simulink model "fft_core"  (Simulink 8.0) (continued)
== system fft_core/fft_wideband_real/fft_direct == (continued)
  butterfly3_2 : Subsystem  AncestorBlock=casper_library_ffts/butterfly_direct  mask(butterfly_direct){n_inputs=1, biplex=off, FFTSize=15, Coeffs=[ 2 8194 4098 12290 2050 10242 6146 14338 1026 9218 5122 13314 3074 11266 7170 15362 514 8706 4610 12802 2562 10754 6658 14850 1538 9730 5634 13826 3586 11778 7682 15874 258 8450 4354 12546 2306 10498 6402 14594 1282 9474 5378 13570 3330 11522 7426 15618 770 8962 4866 13058 2818 11010 6914 15106 1794 9986 5890 14082 3842 12034 7938 16130 130 8322 4226 12418 2178 10370 6274 14466 1154 9346 5250 13442 3202 11394 7298 15490 642 8834 4738 12930 2690 10882 6786 14978 1666 9858 5762 13954 3714 11906 7810 16002 386 8578 4482 12674 2434 10626 6530 14722 1410 9602 5506 13698 3458 11650 7554 15746 898 9090 4994 13186 2946 11138 7042 15234 1922 10114 6018 14210 3970 12162 8066 16258 66 8258 4162 12354 2114 10306 6210 14402 1090 9282 5186 13378 3138 11330 7234 15426 578 8770 4674 12866 2626 10818 6722 14914 1602 9794 5698 13890 3650 11842 7746 15938 322 8514 4418 12610 2370 10562 6466 14658 1346 9538 5442 13634 3394 11586 7490 15682 834 9026 4930 13122 2882 11074 6978 15170 1858 10050 5954 14146 3906 12098 8002 16194 194 8386 4290 12482 2242 10434 6338 14530 1218 9410 5314 13506 3266 11458 7362 15554 706 8898 4802 12994 2754 10946 6850 15042 1730 9922 5826 14018 3778 11970 7874 16066 450 8642 4546 12738 2498 10690 6594 14786 1474 9666 5570 13762 3522 11714 7618 15810 962 9154 5058 13250 3010 11202 7106 15298 1986 10178 6082 14274 4034 12226 8130 16322 34 8226 4130 12322 2082 10274 6178 14370 1058 9250 5154 13346 3106 11298 7202 15394 546 8738 4642 12834 2594 10786 6690 14882 1570 9762 5666 13858 3618 11810 7714 15906 290 8482 4386 12578 2338 10530 6434 14626 1314 9506 5410 13602 3362 11554 7458 15650 802 8994 4898 13090 2850 11042 6946 15138 1826 10018 5922 14114 3874 12066 7970 16162 162 8354 4258 12450 2210 10402 6306 14498 1186 9378 5282 13474 3234 11426 7330 15522 674 8866 4770 12962 2722 10914 6818 15010 1698 9890 5794 13986 3746 11938 7842 16034 418 8610 4514 12706 2466 10658 6562 14754 1442 9634 5538 13730 3490 11682 7586 15778 930 9122 5026 13218 2978 11170 7074 15266 1954 10146 6050 14242 4002 12194 8098 16290 98 8290 4194 12386 2146 10338 6242 14434 1122 9314 5218 13410 3170 11362 7266 15458 610 8802 4706 12898 2658 10850 6754 14946 1634 9826 5730 13922 3682 11874 7778 15970 354 8546 4450 12642 2402 10594 6498 14690 1378 9570 5474 13666 3426 11618 7522 15714 866 9058 4962 13154 2914 11106 7010 15202 1890 10082 5986 14178 3938 12130 8034 16226 226 8418 4322 12514 2274 10466 6370 14562 1250 9442 5346 13538 3298 11490 7394 15586 738 8930 4834 13026 2786 10978 6882 15074 1762 9954 5858 14050 3810 12002 7906 16098 482 8674 4578 12770 2530 10722 6626 14818 1506 9698 5602 13794 3554 11746 7650 15842 994 9186 5090 13282 3042 11234 7138 15330 2018 10210 6114 14306 4066 12258 8162 16354 18 8210 4114 12306 2066 10258 6162 14354 1042 9234 5138 13330 3090 11282 7186 15378 530 8722 4626 12818 2578 10770 6674 14866 1554 9746 5650 13842 3602 11794 7698 15890 274 8466 4370 12562 2322 10514 6418 14610 1298 9490 5394 13586 3346 11538 7442 15634 786 8978 4882 13074 2834 11026 6930 15122 1810 10002 5906 14098 3858 12050 7954 16146 146 8338 4242 12434 2194 10386 6290 14482 1170 9362 5266 13458 3218 11410 7314 15506 658 8850 4754 12946 2706 10898 6802 14994 1682 9874 5778 13970 3730 11922 7826 16018 402 8594 4498 12690 2450 10642 6546 14738 1426 9618 5522 13714 3474 11666 7570 15762 914 9106 5010 13202 2962 11154 7058 15250 1938 10130 6034 14226 3986 12178 8082 16274 82 8274 4178 12370 2130 10322 6226 14418 1106 9298 5202 13394 3154 11346 7250 15442 594 8786 4690 12882 2642 10834 6738 14930 1618 9810 5714 13906 3666 11858 7762 15954 338 8530 4434 12626 2386 10578 6482 14674 1362 9554 5458 13650 3410 11602 7506 15698 850 9042 4946 13138 2898 11090 6994 15186 1874 10066 5970 14162 3922 12114 8018 16210 210 8402 4306 12498 2258 10450 6354 14546 1234 9426 5330 13522 3282 11474 7378 15570 722 8914 4818 13010 2770 10962 6866 15058 1746 9938 5842 14034 3794 11986 7890 16082 466 8658 4562 12754 2514 10706 6610 14802 1490 9682 5586 13778 3538 11730 7634 15826 ...(+6901 chars)], StepPeriod=0, coeff_bit_width=18, input_bit_width=18, bin_pt_in=17, downshift=off, async=off, add_latency=3, mult_latency=3, bram_latency=5, conv_latency=3, quantization=Round  (unbiased: +/- Inf), overflow=Wrap, coeffs_bit_limit=6, coeff_sharing=on, coeff_decimation=off, coeff_generation=off, cal_bits=cal_bits, n_bits_rotation=n_bits_rotation, max_fanout=max_fanout, use_hdl=off, use_embedded=on, bitgrowth=off, hardcode_shifts=off, dsp48_adders=off}
      mask prompts: n_inputs "number inputs"; biplex "Used in biplex FFT"; FFTSize "Size of FFT: (2^? pts)"; Coeffs "Coefficients: (0 to 2^(FFTSize-1)-1)"; StepPeriod "Coefficient Step Period: (2^?)"; coeff_bit_width "Coefficient Bit Width"; input_bit_width "Input Bit Width"; bin_pt_in "Input binary point"; async "asynchronous operation"; add_latency "Add Latency"; mult_latency "Mult Latency"; bram_latency "BRAM Latency"; conv_latency "Convert latency"; quantization "Quantization Behavior"; overflow "Overflow Behavior"; coeffs_bit_limit "Number bits above which to store stage's coefficients in BRAM (2^? bits)"; coeff_sharing "BRAM sharing in coeff storage"; coeff_decimation "Store a fraction of coeff factors where useful"; coeff_generation "Generate coeffs with multipliers where useful"; cal_bits "Number calibration locations when generating coeffs (2^?)"; n_bits_rotation "Feedback rotation vector resolution"; max_fanout "Maximum fanout"; use_hdl "Use behavioural HDL for multipliers"; use_embedded "Use embedded multipliers"; bitgrowth "Bit growth instead of shifting"; hardcode_shifts "Hardcode shift schedule"; dsp48_adders "Use DSP48s for adders"
      mask help: FFT internal block.
      mask init: same as fft_core/fft_wideband_real/fft_direct/butterfly0_0
      settings: AttributesFormatString=twiddle_general
      mask TypeOptions quantization: Truncate | Round  (unbiased: +/- Inf) | Round  (unbiased: Even Values)
      mask TypeOptions overflow: Wrap | Saturate | Error
      mask Callback use_hdl:
      | % make mutually exclusive with use_embedded.
      | 
      | use_hdl = get_param(gcb, 'use_hdl');
      | if strcmp(use_hdl, 'on'),
      |     set_param_state(gcb, 'use_embedded', 'off');
      | else
      |     set_param_state(gcb, 'use_embedded', 'on');
      | end
      mask Callback use_embedded:
      | % make mutually exclusive with use_hdl.
      | 
      | use_hdl = get_param(gcb, 'use_embedded');
      | if strcmp(use_hdl, 'on'),
      |     set_param_state(gcb, 'use_hdl', 'off');
      | else
      |     set_param_state(gcb, 'use_hdl', 'on');
      | end
      mask Callback hardcode_shifts:
      | hardcode_shifts = get_param(gcb, 'hardcode_shifts');
      | set_param_state(gcb, 'downshift', hardcode_shifts);
      mask Callback dsp48_adders: %fft_callback_dsp48_adders(gcb);
  butterfly3_3 : Subsystem  AncestorBlock=casper_library_ffts/butterfly_direct  mask(butterfly_direct){n_inputs=1, biplex=off, FFTSize=15, Coeffs=[ 3 8195 4099 12291 2051 10243 6147 14339 1027 9219 5123 13315 3075 11267 7171 15363 515 8707 4611 12803 2563 10755 6659 14851 1539 9731 5635 13827 3587 11779 7683 15875 259 8451 4355 12547 2307 10499 6403 14595 1283 9475 5379 13571 3331 11523 7427 15619 771 8963 4867 13059 2819 11011 6915 15107 1795 9987 5891 14083 3843 12035 7939 16131 131 8323 4227 12419 2179 10371 6275 14467 1155 9347 5251 13443 3203 11395 7299 15491 643 8835 4739 12931 2691 10883 6787 14979 1667 9859 5763 13955 3715 11907 7811 16003 387 8579 4483 12675 2435 10627 6531 14723 1411 9603 5507 13699 3459 11651 7555 15747 899 9091 4995 13187 2947 11139 7043 15235 1923 10115 6019 14211 3971 12163 8067 16259 67 8259 4163 12355 2115 10307 6211 14403 1091 9283 5187 13379 3139 11331 7235 15427 579 8771 4675 12867 2627 10819 6723 14915 1603 9795 5699 13891 3651 11843 7747 15939 323 8515 4419 12611 2371 10563 6467 14659 1347 9539 5443 13635 3395 11587 7491 15683 835 9027 4931 13123 2883 11075 6979 15171 1859 10051 5955 14147 3907 12099 8003 16195 195 8387 4291 12483 2243 10435 6339 14531 1219 9411 5315 13507 3267 11459 7363 15555 707 8899 4803 12995 2755 10947 6851 15043 1731 9923 5827 14019 3779 11971 7875 16067 451 8643 4547 12739 2499 10691 6595 14787 1475 9667 5571 13763 3523 11715 7619 15811 963 9155 5059 13251 3011 11203 7107 15299 1987 10179 6083 14275 4035 12227 8131 16323 35 8227 4131 12323 2083 10275 6179 14371 1059 9251 5155 13347 3107 11299 7203 15395 547 8739 4643 12835 2595 10787 6691 14883 1571 9763 5667 13859 3619 11811 7715 15907 291 8483 4387 12579 2339 10531 6435 14627 1315 9507 5411 13603 3363 11555 7459 15651 803 8995 4899 13091 2851 11043 6947 15139 1827 10019 5923 14115 3875 12067 7971 16163 163 8355 4259 12451 2211 10403 6307 14499 1187 9379 5283 13475 3235 11427 7331 15523 675 8867 4771 12963 2723 10915 6819 15011 1699 9891 5795 13987 3747 11939 7843 16035 419 8611 4515 12707 2467 10659 6563 14755 1443 9635 5539 13731 3491 11683 7587 15779 931 9123 5027 13219 2979 11171 7075 15267 1955 10147 6051 14243 4003 12195 8099 16291 99 8291 4195 12387 2147 10339 6243 14435 1123 9315 5219 13411 3171 11363 7267 15459 611 8803 4707 12899 2659 10851 6755 14947 1635 9827 5731 13923 3683 11875 7779 15971 355 8547 4451 12643 2403 10595 6499 14691 1379 9571 5475 13667 3427 11619 7523 15715 867 9059 4963 13155 2915 11107 7011 15203 1891 10083 5987 14179 3939 12131 8035 16227 227 8419 4323 12515 2275 10467 6371 14563 1251 9443 5347 13539 3299 11491 7395 15587 739 8931 4835 13027 2787 10979 6883 15075 1763 9955 5859 14051 3811 12003 7907 16099 483 8675 4579 12771 2531 10723 6627 14819 1507 9699 5603 13795 3555 11747 7651 15843 995 9187 5091 13283 3043 11235 7139 15331 2019 10211 6115 14307 4067 12259 8163 16355 19 8211 4115 12307 2067 10259 6163 14355 1043 9235 5139 13331 3091 11283 7187 15379 531 8723 4627 12819 2579 10771 6675 14867 1555 9747 5651 13843 3603 11795 7699 15891 275 8467 4371 12563 2323 10515 6419 14611 1299 9491 5395 13587 3347 11539 7443 15635 787 8979 4883 13075 2835 11027 6931 15123 1811 10003 5907 14099 3859 12051 7955 16147 147 8339 4243 12435 2195 10387 6291 14483 1171 9363 5267 13459 3219 11411 7315 15507 659 8851 4755 12947 2707 10899 6803 14995 1683 9875 5779 13971 3731 11923 7827 16019 403 8595 4499 12691 2451 10643 6547 14739 1427 9619 5523 13715 3475 11667 7571 15763 915 9107 5011 13203 2963 11155 7059 15251 1939 10131 6035 14227 3987 12179 8083 16275 83 8275 4179 12371 2131 10323 6227 14419 1107 9299 5203 13395 3155 11347 7251 15443 595 8787 4691 12883 2643 10835 6739 14931 1619 9811 5715 13907 3667 11859 7763 15955 339 8531 4435 12627 2387 10579 6483 14675 1363 9555 5459 13651 3411 11603 7507 15699 851 9043 4947 13139 2899 11091 6995 15187 1875 10067 5971 14163 3923 12115 8019 16211 211 8403 4307 12499 2259 10451 6355 14547 1235 9427 5331 13523 3283 11475 7379 15571 723 8915 4819 13011 2771 10963 6867 15059 1747 9939 5843 14035 3795 11987 7891 16083 467 8659 4563 12755 2515 10707 6611 14803 1491 9683 5587 13779 3539 11731 7635 15827 ...(+6901 chars)], StepPeriod=0, coeff_bit_width=18, input_bit_width=18, bin_pt_in=17, downshift=off, async=off, add_latency=3, mult_latency=3, bram_latency=5, conv_latency=3, quantization=Round  (unbiased: +/- Inf), overflow=Wrap, coeffs_bit_limit=6, coeff_sharing=on, coeff_decimation=off, coeff_generation=off, cal_bits=cal_bits, n_bits_rotation=n_bits_rotation, max_fanout=max_fanout, use_hdl=off, use_embedded=on, bitgrowth=off, hardcode_shifts=off, dsp48_adders=off}
      mask prompts: n_inputs "number inputs"; biplex "Used in biplex FFT"; FFTSize "Size of FFT: (2^? pts)"; Coeffs "Coefficients: (0 to 2^(FFTSize-1)-1)"; StepPeriod "Coefficient Step Period: (2^?)"; coeff_bit_width "Coefficient Bit Width"; input_bit_width "Input Bit Width"; bin_pt_in "Input binary point"; async "asynchronous operation"; add_latency "Add Latency"; mult_latency "Mult Latency"; bram_latency "BRAM Latency"; conv_latency "Convert latency"; quantization "Quantization Behavior"; overflow "Overflow Behavior"; coeffs_bit_limit "Number bits above which to store stage's coefficients in BRAM (2^? bits)"; coeff_sharing "BRAM sharing in coeff storage"; coeff_decimation "Store a fraction of coeff factors where useful"; coeff_generation "Generate coeffs with multipliers where useful"; cal_bits "Number calibration locations when generating coeffs (2^?)"; n_bits_rotation "Feedback rotation vector resolution"; max_fanout "Maximum fanout"; use_hdl "Use behavioural HDL for multipliers"; use_embedded "Use embedded multipliers"; bitgrowth "Bit growth instead of shifting"; hardcode_shifts "Hardcode shift schedule"; dsp48_adders "Use DSP48s for adders"
      mask help: FFT internal block.
      mask init: same as fft_core/fft_wideband_real/fft_direct/butterfly0_0
      settings: AttributesFormatString=twiddle_general
      mask TypeOptions quantization: Truncate | Round  (unbiased: +/- Inf) | Round  (unbiased: Even Values)
      mask TypeOptions overflow: Wrap | Saturate | Error
      mask Callback use_hdl:
      | % make mutually exclusive with use_embedded.
      | 
      | use_hdl = get_param(gcb, 'use_hdl');
      | if strcmp(use_hdl, 'on'),
      |     set_param_state(gcb, 'use_embedded', 'off');
      | else
      |     set_param_state(gcb, 'use_embedded', 'on');
      | end
      mask Callback use_embedded:
      | % make mutually exclusive with use_hdl.
      | 
      | use_hdl = get_param(gcb, 'use_embedded');
      | if strcmp(use_hdl, 'on'),
      |     set_param_state(gcb, 'use_hdl', 'off');
      | else
      |     set_param_state(gcb, 'use_hdl', 'on');
      | end
      mask Callback hardcode_shifts:
      | hardcode_shifts = get_param(gcb, 'hardcode_shifts');
      | set_param_state(gcb, 'downshift', hardcode_shifts);
      mask Callback dsp48_adders: %fft_callback_dsp48_adders(gcb);
  butterfly3_4 : Subsystem  AncestorBlock=casper_library_ffts/butterfly_direct  mask(butterfly_direct){n_inputs=1, biplex=off, FFTSize=15, Coeffs=[ 4 8196 4100 12292 2052 10244 6148 14340 1028 9220 5124 13316 3076 11268 7172 15364 516 8708 4612 12804 2564 10756 6660 14852 1540 9732 5636 13828 3588 11780 7684 15876 260 8452 4356 12548 2308 10500 6404 14596 1284 9476 5380 13572 3332 11524 7428 15620 772 8964 4868 13060 2820 11012 6916 15108 1796 9988 5892 14084 3844 12036 7940 16132 132 8324 4228 12420 2180 10372 6276 14468 1156 9348 5252 13444 3204 11396 7300 15492 644 8836 4740 12932 2692 10884 6788 14980 1668 9860 5764 13956 3716 11908 7812 16004 388 8580 4484 12676 2436 10628 6532 14724 1412 9604 5508 13700 3460 11652 7556 15748 900 9092 4996 13188 2948 11140 7044 15236 1924 10116 6020 14212 3972 12164 8068 16260 68 8260 4164 12356 2116 10308 6212 14404 1092 9284 5188 13380 3140 11332 7236 15428 580 8772 4676 12868 2628 10820 6724 14916 1604 9796 5700 13892 3652 11844 7748 15940 324 8516 4420 12612 2372 10564 6468 14660 1348 9540 5444 13636 3396 11588 7492 15684 836 9028 4932 13124 2884 11076 6980 15172 1860 10052 5956 14148 3908 12100 8004 16196 196 8388 4292 12484 2244 10436 6340 14532 1220 9412 5316 13508 3268 11460 7364 15556 708 8900 4804 12996 2756 10948 6852 15044 1732 9924 5828 14020 3780 11972 7876 16068 452 8644 4548 12740 2500 10692 6596 14788 1476 9668 5572 13764 3524 11716 7620 15812 964 9156 5060 13252 3012 11204 7108 15300 1988 10180 6084 14276 4036 12228 8132 16324 36 8228 4132 12324 2084 10276 6180 14372 1060 9252 5156 13348 3108 11300 7204 15396 548 8740 4644 12836 2596 10788 6692 14884 1572 9764 5668 13860 3620 11812 7716 15908 292 8484 4388 12580 2340 10532 6436 14628 1316 9508 5412 13604 3364 11556 7460 15652 804 8996 4900 13092 2852 11044 6948 15140 1828 10020 5924 14116 3876 12068 7972 16164 164 8356 4260 12452 2212 10404 6308 14500 1188 9380 5284 13476 3236 11428 7332 15524 676 8868 4772 12964 2724 10916 6820 15012 1700 9892 5796 13988 3748 11940 7844 16036 420 8612 4516 12708 2468 10660 6564 14756 1444 9636 5540 13732 3492 11684 7588 15780 932 9124 5028 13220 2980 11172 7076 15268 1956 10148 6052 14244 4004 12196 8100 16292 100 8292 4196 12388 2148 10340 6244 14436 1124 9316 5220 13412 3172 11364 7268 15460 612 8804 4708 12900 2660 10852 6756 14948 1636 9828 5732 13924 3684 11876 7780 15972 356 8548 4452 12644 2404 10596 6500 14692 1380 9572 5476 13668 3428 11620 7524 15716 868 9060 4964 13156 2916 11108 7012 15204 1892 10084 5988 14180 3940 12132 8036 16228 228 8420 4324 12516 2276 10468 6372 14564 1252 9444 5348 13540 3300 11492 7396 15588 740 8932 4836 13028 2788 10980 6884 15076 1764 9956 5860 14052 3812 12004 7908 16100 484 8676 4580 12772 2532 10724 6628 14820 1508 9700 5604 13796 3556 11748 7652 15844 996 9188 5092 13284 3044 11236 7140 15332 2020 10212 6116 14308 4068 12260 8164 16356 20 8212 4116 12308 2068 10260 6164 14356 1044 9236 5140 13332 3092 11284 7188 15380 532 8724 4628 12820 2580 10772 6676 14868 1556 9748 5652 13844 3604 11796 7700 15892 276 8468 4372 12564 2324 10516 6420 14612 1300 9492 5396 13588 3348 11540 7444 15636 788 8980 4884 13076 2836 11028 6932 15124 1812 10004 5908 14100 3860 12052 7956 16148 148 8340 4244 12436 2196 10388 6292 14484 1172 9364 5268 13460 3220 11412 7316 15508 660 8852 4756 12948 2708 10900 6804 14996 1684 9876 5780 13972 3732 11924 7828 16020 404 8596 4500 12692 2452 10644 6548 14740 1428 9620 5524 13716 3476 11668 7572 15764 916 9108 5012 13204 2964 11156 7060 15252 1940 10132 6036 14228 3988 12180 8084 16276 84 8276 4180 12372 2132 10324 6228 14420 1108 9300 5204 13396 3156 11348 7252 15444 596 8788 4692 12884 2644 10836 6740 14932 1620 9812 5716 13908 3668 11860 7764 15956 340 8532 4436 12628 2388 10580 6484 14676 1364 9556 5460 13652 3412 11604 7508 15700 852 9044 4948 13140 2900 11092 6996 15188 1876 10068 5972 14164 3924 12116 8020 16212 212 8404 4308 12500 2260 10452 6356 14548 1236 9428 5332 13524 3284 11476 7380 15572 724 8916 4820 13012 2772 10964 6868 15060 1748 9940 5844 14036 3796 11988 7892 16084 468 8660 4564 12756 2516 10708 6612 14804 1492 9684 5588 13780 3540 11732 7636 ...(+6907 chars)], StepPeriod=0, coeff_bit_width=18, input_bit_width=18, bin_pt_in=17, downshift=off, async=off, add_latency=3, mult_latency=3, bram_latency=5, conv_latency=3, quantization=Round  (unbiased: +/- Inf), overflow=Wrap, coeffs_bit_limit=6, coeff_sharing=on, coeff_decimation=off, coeff_generation=off, cal_bits=cal_bits, n_bits_rotation=n_bits_rotation, max_fanout=max_fanout, use_hdl=off, use_embedded=on, bitgrowth=off, hardcode_shifts=off, dsp48_adders=off}
      mask prompts: n_inputs "number inputs"; biplex "Used in biplex FFT"; FFTSize "Size of FFT: (2^? pts)"; Coeffs "Coefficients: (0 to 2^(FFTSize-1)-1)"; StepPeriod "Coefficient Step Period: (2^?)"; coeff_bit_width "Coefficient Bit Width"; input_bit_width "Input Bit Width"; bin_pt_in "Input binary point"; async "asynchronous operation"; add_latency "Add Latency"; mult_latency "Mult Latency"; bram_latency "BRAM Latency"; conv_latency "Convert latency"; quantization "Quantization Behavior"; overflow "Overflow Behavior"; coeffs_bit_limit "Number bits above which to store stage's coefficients in BRAM (2^? bits)"; coeff_sharing "BRAM sharing in coeff storage"; coeff_decimation "Store a fraction of coeff factors where useful"; coeff_generation "Generate coeffs with multipliers where useful"; cal_bits "Number calibration locations when generating coeffs (2^?)"; n_bits_rotation "Feedback rotation vector resolution"; max_fanout "Maximum fanout"; use_hdl "Use behavioural HDL for multipliers"; use_embedded "Use embedded multipliers"; bitgrowth "Bit growth instead of shifting"; hardcode_shifts "Hardcode shift schedule"; dsp48_adders "Use DSP48s for adders"
      mask help: FFT internal block.
      mask init: same as fft_core/fft_wideband_real/fft_direct/butterfly0_0
      settings: AttributesFormatString=twiddle_general
      mask TypeOptions quantization: Truncate | Round  (unbiased: +/- Inf) | Round  (unbiased: Even Values)
      mask TypeOptions overflow: Wrap | Saturate | Error
      mask Callback use_hdl:
      | % make mutually exclusive with use_embedded.
      | 
      | use_hdl = get_param(gcb, 'use_hdl');
      | if strcmp(use_hdl, 'on'),
      |     set_param_state(gcb, 'use_embedded', 'off');
      | else
      |     set_param_state(gcb, 'use_embedded', 'on');
      | end
      mask Callback use_embedded:
      | % make mutually exclusive with use_hdl.
      | 
      | use_hdl = get_param(gcb, 'use_embedded');
      | if strcmp(use_hdl, 'on'),
      |     set_param_state(gcb, 'use_hdl', 'off');
      | else
      |     set_param_state(gcb, 'use_hdl', 'on');
      | end
      mask Callback hardcode_shifts:
      | hardcode_shifts = get_param(gcb, 'hardcode_shifts');
      | set_param_state(gcb, 'downshift', hardcode_shifts);
      mask Callback dsp48_adders: %fft_callback_dsp48_adders(gcb);
  butterfly3_5 : Subsystem  AncestorBlock=casper_library_ffts/butterfly_direct  mask(butterfly_direct){n_inputs=1, biplex=off, FFTSize=15, Coeffs=[ 5 8197 4101 12293 2053 10245 6149 14341 1029 9221 5125 13317 3077 11269 7173 15365 517 8709 4613 12805 2565 10757 6661 14853 1541 9733 5637 13829 3589 11781 7685 15877 261 8453 4357 12549 2309 10501 6405 14597 1285 9477 5381 13573 3333 11525 7429 15621 773 8965 4869 13061 2821 11013 6917 15109 1797 9989 5893 14085 3845 12037 7941 16133 133 8325 4229 12421 2181 10373 6277 14469 1157 9349 5253 13445 3205 11397 7301 15493 645 8837 4741 12933 2693 10885 6789 14981 1669 9861 5765 13957 3717 11909 7813 16005 389 8581 4485 12677 2437 10629 6533 14725 1413 9605 5509 13701 3461 11653 7557 15749 901 9093 4997 13189 2949 11141 7045 15237 1925 10117 6021 14213 3973 12165 8069 16261 69 8261 4165 12357 2117 10309 6213 14405 1093 9285 5189 13381 3141 11333 7237 15429 581 8773 4677 12869 2629 10821 6725 14917 1605 9797 5701 13893 3653 11845 7749 15941 325 8517 4421 12613 2373 10565 6469 14661 1349 9541 5445 13637 3397 11589 7493 15685 837 9029 4933 13125 2885 11077 6981 15173 1861 10053 5957 14149 3909 12101 8005 16197 197 8389 4293 12485 2245 10437 6341 14533 1221 9413 5317 13509 3269 11461 7365 15557 709 8901 4805 12997 2757 10949 6853 15045 1733 9925 5829 14021 3781 11973 7877 16069 453 8645 4549 12741 2501 10693 6597 14789 1477 9669 5573 13765 3525 11717 7621 15813 965 9157 5061 13253 3013 11205 7109 15301 1989 10181 6085 14277 4037 12229 8133 16325 37 8229 4133 12325 2085 10277 6181 14373 1061 9253 5157 13349 3109 11301 7205 15397 549 8741 4645 12837 2597 10789 6693 14885 1573 9765 5669 13861 3621 11813 7717 15909 293 8485 4389 12581 2341 10533 6437 14629 1317 9509 5413 13605 3365 11557 7461 15653 805 8997 4901 13093 2853 11045 6949 15141 1829 10021 5925 14117 3877 12069 7973 16165 165 8357 4261 12453 2213 10405 6309 14501 1189 9381 5285 13477 3237 11429 7333 15525 677 8869 4773 12965 2725 10917 6821 15013 1701 9893 5797 13989 3749 11941 7845 16037 421 8613 4517 12709 2469 10661 6565 14757 1445 9637 5541 13733 3493 11685 7589 15781 933 9125 5029 13221 2981 11173 7077 15269 1957 10149 6053 14245 4005 12197 8101 16293 101 8293 4197 12389 2149 10341 6245 14437 1125 9317 5221 13413 3173 11365 7269 15461 613 8805 4709 12901 2661 10853 6757 14949 1637 9829 5733 13925 3685 11877 7781 15973 357 8549 4453 12645 2405 10597 6501 14693 1381 9573 5477 13669 3429 11621 7525 15717 869 9061 4965 13157 2917 11109 7013 15205 1893 10085 5989 14181 3941 12133 8037 16229 229 8421 4325 12517 2277 10469 6373 14565 1253 9445 5349 13541 3301 11493 7397 15589 741 8933 4837 13029 2789 10981 6885 15077 1765 9957 5861 14053 3813 12005 7909 16101 485 8677 4581 12773 2533 10725 6629 14821 1509 9701 5605 13797 3557 11749 7653 15845 997 9189 5093 13285 3045 11237 7141 15333 2021 10213 6117 14309 4069 12261 8165 16357 21 8213 4117 12309 2069 10261 6165 14357 1045 9237 5141 13333 3093 11285 7189 15381 533 8725 4629 12821 2581 10773 6677 14869 1557 9749 5653 13845 3605 11797 7701 15893 277 8469 4373 12565 2325 10517 6421 14613 1301 9493 5397 13589 3349 11541 7445 15637 789 8981 4885 13077 2837 11029 6933 15125 1813 10005 5909 14101 3861 12053 7957 16149 149 8341 4245 12437 2197 10389 6293 14485 1173 9365 5269 13461 3221 11413 7317 15509 661 8853 4757 12949 2709 10901 6805 14997 1685 9877 5781 13973 3733 11925 7829 16021 405 8597 4501 12693 2453 10645 6549 14741 1429 9621 5525 13717 3477 11669 7573 15765 917 9109 5013 13205 2965 11157 7061 15253 1941 10133 6037 14229 3989 12181 8085 16277 85 8277 4181 12373 2133 10325 6229 14421 1109 9301 5205 13397 3157 11349 7253 15445 597 8789 4693 12885 2645 10837 6741 14933 1621 9813 5717 13909 3669 11861 7765 15957 341 8533 4437 12629 2389 10581 6485 14677 1365 9557 5461 13653 3413 11605 7509 15701 853 9045 4949 13141 2901 11093 6997 15189 1877 10069 5973 14165 3925 12117 8021 16213 213 8405 4309 12501 2261 10453 6357 14549 1237 9429 5333 13525 3285 11477 7381 15573 725 8917 4821 13013 2773 10965 6869 15061 1749 9941 5845 14037 3797 11989 7893 16085 469 8661 4565 12757 2517 10709 6613 14805 1493 9685 5589 13781 3541 11733 7637 ...(+6907 chars)], StepPeriod=0, coeff_bit_width=18, input_bit_width=18, bin_pt_in=17, downshift=off, async=off, add_latency=3, mult_latency=3, bram_latency=5, conv_latency=3, quantization=Round  (unbiased: +/- Inf), overflow=Wrap, coeffs_bit_limit=6, coeff_sharing=on, coeff_decimation=off, coeff_generation=off, cal_bits=cal_bits, n_bits_rotation=n_bits_rotation, max_fanout=max_fanout, use_hdl=off, use_embedded=on, bitgrowth=off, hardcode_shifts=off, dsp48_adders=off}
      mask prompts: n_inputs "number inputs"; biplex "Used in biplex FFT"; FFTSize "Size of FFT: (2^? pts)"; Coeffs "Coefficients: (0 to 2^(FFTSize-1)-1)"; StepPeriod "Coefficient Step Period: (2^?)"; coeff_bit_width "Coefficient Bit Width"; input_bit_width "Input Bit Width"; bin_pt_in "Input binary point"; async "asynchronous operation"; add_latency "Add Latency"; mult_latency "Mult Latency"; bram_latency "BRAM Latency"; conv_latency "Convert latency"; quantization "Quantization Behavior"; overflow "Overflow Behavior"; coeffs_bit_limit "Number bits above which to store stage's coefficients in BRAM (2^? bits)"; coeff_sharing "BRAM sharing in coeff storage"; coeff_decimation "Store a fraction of coeff factors where useful"; coeff_generation "Generate coeffs with multipliers where useful"; cal_bits "Number calibration locations when generating coeffs (2^?)"; n_bits_rotation "Feedback rotation vector resolution"; max_fanout "Maximum fanout"; use_hdl "Use behavioural HDL for multipliers"; use_embedded "Use embedded multipliers"; bitgrowth "Bit growth instead of shifting"; hardcode_shifts "Hardcode shift schedule"; dsp48_adders "Use DSP48s for adders"
      mask help: FFT internal block.
      mask init: same as fft_core/fft_wideband_real/fft_direct/butterfly0_0
      settings: AttributesFormatString=twiddle_general
      mask TypeOptions quantization: Truncate | Round  (unbiased: +/- Inf) | Round  (unbiased: Even Values)
      mask TypeOptions overflow: Wrap | Saturate | Error
      mask Callback use_hdl:
      | % make mutually exclusive with use_embedded.
      | 
      | use_hdl = get_param(gcb, 'use_hdl');
      | if strcmp(use_hdl, 'on'),
      |     set_param_state(gcb, 'use_embedded', 'off');
      | else
      |     set_param_state(gcb, 'use_embedded', 'on');
      | end
      mask Callback use_embedded:
      | % make mutually exclusive with use_hdl.
      | 
      | use_hdl = get_param(gcb, 'use_embedded');
      | if strcmp(use_hdl, 'on'),
      |     set_param_state(gcb, 'use_hdl', 'off');
      | else
      |     set_param_state(gcb, 'use_hdl', 'on');
      | end
      mask Callback hardcode_shifts:
      | hardcode_shifts = get_param(gcb, 'hardcode_shifts');
      | set_param_state(gcb, 'downshift', hardcode_shifts);
      mask Callback dsp48_adders: %fft_callback_dsp48_adders(gcb);
  butterfly3_6 : Subsystem  AncestorBlock=casper_library_ffts/butterfly_direct  mask(butterfly_direct){n_inputs=1, biplex=off, FFTSize=15, Coeffs=[ 6 8198 4102 12294 2054 10246 6150 14342 1030 9222 5126 13318 3078 11270 7174 15366 518 8710 4614 12806 2566 10758 6662 14854 1542 9734 5638 13830 3590 11782 7686 15878 262 8454 4358 12550 2310 10502 6406 14598 1286 9478 5382 13574 3334 11526 7430 15622 774 8966 4870 13062 2822 11014 6918 15110 1798 9990 5894 14086 3846 12038 7942 16134 134 8326 4230 12422 2182 10374 6278 14470 1158 9350 5254 13446 3206 11398 7302 15494 646 8838 4742 12934 2694 10886 6790 14982 1670 9862 5766 13958 3718 11910 7814 16006 390 8582 4486 12678 2438 10630 6534 14726 1414 9606 5510 13702 3462 11654 7558 15750 902 9094 4998 13190 2950 11142 7046 15238 1926 10118 6022 14214 3974 12166 8070 16262 70 8262 4166 12358 2118 10310 6214 14406 1094 9286 5190 13382 3142 11334 7238 15430 582 8774 4678 12870 2630 10822 6726 14918 1606 9798 5702 13894 3654 11846 7750 15942 326 8518 4422 12614 2374 10566 6470 14662 1350 9542 5446 13638 3398 11590 7494 15686 838 9030 4934 13126 2886 11078 6982 15174 1862 10054 5958 14150 3910 12102 8006 16198 198 8390 4294 12486 2246 10438 6342 14534 1222 9414 5318 13510 3270 11462 7366 15558 710 8902 4806 12998 2758 10950 6854 15046 1734 9926 5830 14022 3782 11974 7878 16070 454 8646 4550 12742 2502 10694 6598 14790 1478 9670 5574 13766 3526 11718 7622 15814 966 9158 5062 13254 3014 11206 7110 15302 1990 10182 6086 14278 4038 12230 8134 16326 38 8230 4134 12326 2086 10278 6182 14374 1062 9254 5158 13350 3110 11302 7206 15398 550 8742 4646 12838 2598 10790 6694 14886 1574 9766 5670 13862 3622 11814 7718 15910 294 8486 4390 12582 2342 10534 6438 14630 1318 9510 5414 13606 3366 11558 7462 15654 806 8998 4902 13094 2854 11046 6950 15142 1830 10022 5926 14118 3878 12070 7974 16166 166 8358 4262 12454 2214 10406 6310 14502 1190 9382 5286 13478 3238 11430 7334 15526 678 8870 4774 12966 2726 10918 6822 15014 1702 9894 5798 13990 3750 11942 7846 16038 422 8614 4518 12710 2470 10662 6566 14758 1446 9638 5542 13734 3494 11686 7590 15782 934 9126 5030 13222 2982 11174 7078 15270 1958 10150 6054 14246 4006 12198 8102 16294 102 8294 4198 12390 2150 10342 6246 14438 1126 9318 5222 13414 3174 11366 7270 15462 614 8806 4710 12902 2662 10854 6758 14950 1638 9830 5734 13926 3686 11878 7782 15974 358 8550 4454 12646 2406 10598 6502 14694 1382 9574 5478 13670 3430 11622 7526 15718 870 9062 4966 13158 2918 11110 7014 15206 1894 10086 5990 14182 3942 12134 8038 16230 230 8422 4326 12518 2278 10470 6374 14566 1254 9446 5350 13542 3302 11494 7398 15590 742 8934 4838 13030 2790 10982 6886 15078 1766 9958 5862 14054 3814 12006 7910 16102 486 8678 4582 12774 2534 10726 6630 14822 1510 9702 5606 13798 3558 11750 7654 15846 998 9190 5094 13286 3046 11238 7142 15334 2022 10214 6118 14310 4070 12262 8166 16358 22 8214 4118 12310 2070 10262 6166 14358 1046 9238 5142 13334 3094 11286 7190 15382 534 8726 4630 12822 2582 10774 6678 14870 1558 9750 5654 13846 3606 11798 7702 15894 278 8470 4374 12566 2326 10518 6422 14614 1302 9494 5398 13590 3350 11542 7446 15638 790 8982 4886 13078 2838 11030 6934 15126 1814 10006 5910 14102 3862 12054 7958 16150 150 8342 4246 12438 2198 10390 6294 14486 1174 9366 5270 13462 3222 11414 7318 15510 662 8854 4758 12950 2710 10902 6806 14998 1686 9878 5782 13974 3734 11926 7830 16022 406 8598 4502 12694 2454 10646 6550 14742 1430 9622 5526 13718 3478 11670 7574 15766 918 9110 5014 13206 2966 11158 7062 15254 1942 10134 6038 14230 3990 12182 8086 16278 86 8278 4182 12374 2134 10326 6230 14422 1110 9302 5206 13398 3158 11350 7254 15446 598 8790 4694 12886 2646 10838 6742 14934 1622 9814 5718 13910 3670 11862 7766 15958 342 8534 4438 12630 2390 10582 6486 14678 1366 9558 5462 13654 3414 11606 7510 15702 854 9046 4950 13142 2902 11094 6998 15190 1878 10070 5974 14166 3926 12118 8022 16214 214 8406 4310 12502 2262 10454 6358 14550 1238 9430 5334 13526 3286 11478 7382 15574 726 8918 4822 13014 2774 10966 6870 15062 1750 9942 5846 14038 3798 11990 7894 16086 470 8662 4566 12758 2518 10710 6614 14806 1494 9686 5590 13782 3542 11734 7638 ...(+6907 chars)], StepPeriod=0, coeff_bit_width=18, input_bit_width=18, bin_pt_in=17, downshift=off, async=off, add_latency=3, mult_latency=3, bram_latency=5, conv_latency=3, quantization=Round  (unbiased: +/- Inf), overflow=Wrap, coeffs_bit_limit=6, coeff_sharing=on, coeff_decimation=off, coeff_generation=off, cal_bits=cal_bits, n_bits_rotation=n_bits_rotation, max_fanout=max_fanout, use_hdl=off, use_embedded=on, bitgrowth=off, hardcode_shifts=off, dsp48_adders=off}
      mask prompts: n_inputs "number inputs"; biplex "Used in biplex FFT"; FFTSize "Size of FFT: (2^? pts)"; Coeffs "Coefficients: (0 to 2^(FFTSize-1)-1)"; StepPeriod "Coefficient Step Period: (2^?)"; coeff_bit_width "Coefficient Bit Width"; input_bit_width "Input Bit Width"; bin_pt_in "Input binary point"; async "asynchronous operation"; add_latency "Add Latency"; mult_latency "Mult Latency"; bram_latency "BRAM Latency"; conv_latency "Convert latency"; quantization "Quantization Behavior"; overflow "Overflow Behavior"; coeffs_bit_limit "Number bits above which to store stage's coefficients in BRAM (2^? bits)"; coeff_sharing "BRAM sharing in coeff storage"; coeff_decimation "Store a fraction of coeff factors where useful"; coeff_generation "Generate coeffs with multipliers where useful"; cal_bits "Number calibration locations when generating coeffs (2^?)"; n_bits_rotation "Feedback rotation vector resolution"; max_fanout "Maximum fanout"; use_hdl "Use behavioural HDL for multipliers"; use_embedded "Use embedded multipliers"; bitgrowth "Bit growth instead of shifting"; hardcode_shifts "Hardcode shift schedule"; dsp48_adders "Use DSP48s for adders"
      mask help: FFT internal block.
      mask init: same as fft_core/fft_wideband_real/fft_direct/butterfly0_0
      settings: AttributesFormatString=twiddle_general
      mask TypeOptions quantization: Truncate | Round  (unbiased: +/- Inf) | Round  (unbiased: Even Values)
      mask TypeOptions overflow: Wrap | Saturate | Error
      mask Callback use_hdl:
      | % make mutually exclusive with use_embedded.
      | 
      | use_hdl = get_param(gcb, 'use_hdl');
      | if strcmp(use_hdl, 'on'),
      |     set_param_state(gcb, 'use_embedded', 'off');
      | else
      |     set_param_state(gcb, 'use_embedded', 'on');
      | end
      mask Callback use_embedded:
      | % make mutually exclusive with use_hdl.
      | 
      | use_hdl = get_param(gcb, 'use_embedded');
      | if strcmp(use_hdl, 'on'),
      |     set_param_state(gcb, 'use_hdl', 'off');
      | else
      |     set_param_state(gcb, 'use_hdl', 'on');
      | end
      mask Callback hardcode_shifts:
      | hardcode_shifts = get_param(gcb, 'hardcode_shifts');
      | set_param_state(gcb, 'downshift', hardcode_shifts);
      mask Callback dsp48_adders: %fft_callback_dsp48_adders(gcb);
  butterfly3_7 : Subsystem  AncestorBlock=casper_library_ffts/butterfly_direct  mask(butterfly_direct){n_inputs=1, biplex=off, FFTSize=15, Coeffs=[ 7 8199 4103 12295 2055 10247 6151 14343 1031 9223 5127 13319 3079 11271 7175 15367 519 8711 4615 12807 2567 10759 6663 14855 1543 9735 5639 13831 3591 11783 7687 15879 263 8455 4359 12551 2311 10503 6407 14599 1287 9479 5383 13575 3335 11527 7431 15623 775 8967 4871 13063 2823 11015 6919 15111 1799 9991 5895 14087 3847 12039 7943 16135 135 8327 4231 12423 2183 10375 6279 14471 1159 9351 5255 13447 3207 11399 7303 15495 647 8839 4743 12935 2695 10887 6791 14983 1671 9863 5767 13959 3719 11911 7815 16007 391 8583 4487 12679 2439 10631 6535 14727 1415 9607 5511 13703 3463 11655 7559 15751 903 9095 4999 13191 2951 11143 7047 15239 1927 10119 6023 14215 3975 12167 8071 16263 71 8263 4167 12359 2119 10311 6215 14407 1095 9287 5191 13383 3143 11335 7239 15431 583 8775 4679 12871 2631 10823 6727 14919 1607 9799 5703 13895 3655 11847 7751 15943 327 8519 4423 12615 2375 10567 6471 14663 1351 9543 5447 13639 3399 11591 7495 15687 839 9031 4935 13127 2887 11079 6983 15175 1863 10055 5959 14151 3911 12103 8007 16199 199 8391 4295 12487 2247 10439 6343 14535 1223 9415 5319 13511 3271 11463 7367 15559 711 8903 4807 12999 2759 10951 6855 15047 1735 9927 5831 14023 3783 11975 7879 16071 455 8647 4551 12743 2503 10695 6599 14791 1479 9671 5575 13767 3527 11719 7623 15815 967 9159 5063 13255 3015 11207 7111 15303 1991 10183 6087 14279 4039 12231 8135 16327 39 8231 4135 12327 2087 10279 6183 14375 1063 9255 5159 13351 3111 11303 7207 15399 551 8743 4647 12839 2599 10791 6695 14887 1575 9767 5671 13863 3623 11815 7719 15911 295 8487 4391 12583 2343 10535 6439 14631 1319 9511 5415 13607 3367 11559 7463 15655 807 8999 4903 13095 2855 11047 6951 15143 1831 10023 5927 14119 3879 12071 7975 16167 167 8359 4263 12455 2215 10407 6311 14503 1191 9383 5287 13479 3239 11431 7335 15527 679 8871 4775 12967 2727 10919 6823 15015 1703 9895 5799 13991 3751 11943 7847 16039 423 8615 4519 12711 2471 10663 6567 14759 1447 9639 5543 13735 3495 11687 7591 15783 935 9127 5031 13223 2983 11175 7079 15271 1959 10151 6055 14247 4007 12199 8103 16295 103 8295 4199 12391 2151 10343 6247 14439 1127 9319 5223 13415 3175 11367 7271 15463 615 8807 4711 12903 2663 10855 6759 14951 1639 9831 5735 13927 3687 11879 7783 15975 359 8551 4455 12647 2407 10599 6503 14695 1383 9575 5479 13671 3431 11623 7527 15719 871 9063 4967 13159 2919 11111 7015 15207 1895 10087 5991 14183 3943 12135 8039 16231 231 8423 4327 12519 2279 10471 6375 14567 1255 9447 5351 13543 3303 11495 7399 15591 743 8935 4839 13031 2791 10983 6887 15079 1767 9959 5863 14055 3815 12007 7911 16103 487 8679 4583 12775 2535 10727 6631 14823 1511 9703 5607 13799 3559 11751 7655 15847 999 9191 5095 13287 3047 11239 7143 15335 2023 10215 6119 14311 4071 12263 8167 16359 23 8215 4119 12311 2071 10263 6167 14359 1047 9239 5143 13335 3095 11287 7191 15383 535 8727 4631 12823 2583 10775 6679 14871 1559 9751 5655 13847 3607 11799 7703 15895 279 8471 4375 12567 2327 10519 6423 14615 1303 9495 5399 13591 3351 11543 7447 15639 791 8983 4887 13079 2839 11031 6935 15127 1815 10007 5911 14103 3863 12055 7959 16151 151 8343 4247 12439 2199 10391 6295 14487 1175 9367 5271 13463 3223 11415 7319 15511 663 8855 4759 12951 2711 10903 6807 14999 1687 9879 5783 13975 3735 11927 7831 16023 407 8599 4503 12695 2455 10647 6551 14743 1431 9623 5527 13719 3479 11671 7575 15767 919 9111 5015 13207 2967 11159 7063 15255 1943 10135 6039 14231 3991 12183 8087 16279 87 8279 4183 12375 2135 10327 6231 14423 1111 9303 5207 13399 3159 11351 7255 15447 599 8791 4695 12887 2647 10839 6743 14935 1623 9815 5719 13911 3671 11863 7767 15959 343 8535 4439 12631 2391 10583 6487 14679 1367 9559 5463 13655 3415 11607 7511 15703 855 9047 4951 13143 2903 11095 6999 15191 1879 10071 5975 14167 3927 12119 8023 16215 215 8407 4311 12503 2263 10455 6359 14551 1239 9431 5335 13527 3287 11479 7383 15575 727 8919 4823 13015 2775 10967 6871 15063 1751 9943 5847 14039 3799 11991 7895 16087 471 8663 4567 12759 2519 10711 6615 14807 1495 9687 5591 13783 3543 11735 7639 ...(+6907 chars)], StepPeriod=0, coeff_bit_width=18, input_bit_width=18, bin_pt_in=17, downshift=off, async=off, add_latency=3, mult_latency=3, bram_latency=5, conv_latency=3, quantization=Round  (unbiased: +/- Inf), overflow=Wrap, coeffs_bit_limit=6, coeff_sharing=on, coeff_decimation=off, coeff_generation=off, cal_bits=cal_bits, n_bits_rotation=n_bits_rotation, max_fanout=max_fanout, use_hdl=off, use_embedded=on, bitgrowth=off, hardcode_shifts=off, dsp48_adders=off}
      mask prompts: n_inputs "number inputs"; biplex "Used in biplex FFT"; FFTSize "Size of FFT: (2^? pts)"; Coeffs "Coefficients: (0 to 2^(FFTSize-1)-1)"; StepPeriod "Coefficient Step Period: (2^?)"; coeff_bit_width "Coefficient Bit Width"; input_bit_width "Input Bit Width"; bin_pt_in "Input binary point"; async "asynchronous operation"; add_latency "Add Latency"; mult_latency "Mult Latency"; bram_latency "BRAM Latency"; conv_latency "Convert latency"; quantization "Quantization Behavior"; overflow "Overflow Behavior"; coeffs_bit_limit "Number bits above which to store stage's coefficients in BRAM (2^? bits)"; coeff_sharing "BRAM sharing in coeff storage"; coeff_decimation "Store a fraction of coeff factors where useful"; coeff_generation "Generate coeffs with multipliers where useful"; cal_bits "Number calibration locations when generating coeffs (2^?)"; n_bits_rotation "Feedback rotation vector resolution"; max_fanout "Maximum fanout"; use_hdl "Use behavioural HDL for multipliers"; use_embedded "Use embedded multipliers"; bitgrowth "Bit growth instead of shifting"; hardcode_shifts "Hardcode shift schedule"; dsp48_adders "Use DSP48s for adders"
      mask help: FFT internal block.
      mask init: same as fft_core/fft_wideband_real/fft_direct/butterfly0_0
      settings: AttributesFormatString=twiddle_general
      mask TypeOptions quantization: Truncate | Round  (unbiased: +/- Inf) | Round  (unbiased: Even Values)
      mask TypeOptions overflow: Wrap | Saturate | Error
      mask Callback use_hdl:
      | % make mutually exclusive with use_embedded.
      | 
      | use_hdl = get_param(gcb, 'use_hdl');
      | if strcmp(use_hdl, 'on'),
      |     set_param_state(gcb, 'use_embedded', 'off');
      | else
      |     set_param_state(gcb, 'use_embedded', 'on');
      | end
      mask Callback use_embedded:
      | % make mutually exclusive with use_hdl.
      | 
      | use_hdl = get_param(gcb, 'use_embedded');
      | if strcmp(use_hdl, 'on'),
      |     set_param_state(gcb, 'use_hdl', 'off');
      | else
      |     set_param_state(gcb, 'use_hdl', 'on');
      | end
      mask Callback hardcode_shifts:
      | hardcode_shifts = get_param(gcb, 'hardcode_shifts');
      | set_param_state(gcb, 'downshift', hardcode_shifts);
      mask Callback dsp48_adders: %fft_callback_dsp48_adders(gcb);
  combine : xbsIndex_r4/Logical  logical_function=OR  inputs=8  en=off  latency=1  precision=Full  arith_type=Unsigned  n_bits=16  bin_pt=0  align_bp=on  dbl_ovrd=off  block_type=logical
  of_0 : xbsIndex_r4/Delay  rst=off  infoeditControl=Selection of Reset will increase slice count due to use of real FFs and instead of SRLs  en=off  latency=0  dbl_ovrd=off  reg_retiming=on  block_type=delay
  of_1 : xbsIndex_r4/Concat  num_inputs=2  dbl_ovrd=off  block_type=concat
  of_2 : xbsIndex_r4/Concat  num_inputs=4  dbl_ovrd=off  block_type=concat
  of_3 : xbsIndex_r4/Concat  num_inputs=8  dbl_ovrd=off  block_type=concat
  of_expand : Subsystem  AncestorBlock=casper_library_flow_control/bus_expand  mask(bus_expand){mode=divisions of equal size, outputNum=8, outputWidth=1, outputBinaryPt=0, outputArithmeticType=0, show_format=off, outputToWorkspace=off, variablePrefix=out, outputToModelAsWell=on}
      mask prompts: outputNum "Number of outputs:"; outputWidth "Output width:"; outputBinaryPt "Output binary point position:"; outputArithmeticType "Output arithmetic type (ufix=0, fix=1, bool=2):"; show_format "Print format string?"; outputToWorkspace "Output to workspace?"; variablePrefix "Variable name prefix:"; outputToModelAsWell "Output to model as well?"
      mask help:
      | Expands a 'bus' made using a bus_create or Xilinx
      | concat block.
      | The input will be divided into the specified number
      | of outputs with the specified arithmetic types.
      | Divisions are made from most significant bit.
      mask init: same as fft_core/fft_wideband_real/fft_biplex_real_4x/pol0_debus
      settings: AttributesFormatString=8 outputs
      mask TypeOptions mode: divisions of equal size | divisions of arbitrary size
      mask Callback mode: bus_expand_callback();
      mask Callback outputWidth: bus_expand_callback();
      mask Callback outputToWorkspace: bus_expand_callback();
  of_or : xbsIndex_r4/Logical  logical_function=OR  inputs=4  en=off  latency=1  precision=Full  arith_type=Unsigned  n_bits=16  bin_pt=0  align_bp=on  dbl_ovrd=off  block_type=logical
  slice0 : xbsIndex_r4/Slice  nbits=1  boolean_output=on  mode=Lower Bit Location + Width  bit1=0  base1=MSB of Input  bit0=0  base0=LSB of Input  dbl_ovrd=off  block_type=slice
  slice1 : xbsIndex_r4/Slice  nbits=1  boolean_output=on  mode=Lower Bit Location + Width  bit1=0  base1=MSB of Input  bit0=1  base0=LSB of Input  dbl_ovrd=off  block_type=slice
  slice2 : xbsIndex_r4/Slice  nbits=1  boolean_output=on  mode=Lower Bit Location + Width  bit1=0  base1=MSB of Input  bit0=2  base0=LSB of Input  dbl_ovrd=off  block_type=slice
  slice3 : xbsIndex_r4/Slice  nbits=1  boolean_output=on  mode=Lower Bit Location + Width  bit1=0  base1=MSB of Input  bit0=3  base0=LSB of Input  dbl_ovrd=off  block_type=slice
  synct{1..7} (7 alike) : Terminator
  sync_out : Outport
  out00 : Outport  Port=2
  out01 : Outport  Port=3
  out02 : Outport  Port=4
  out03 : Outport  Port=5
  out04 : Outport  Port=6
  out05 : Outport  Port=7
  out06 : Outport  Port=8
  out07 : Outport  Port=9
  out08 : Outport  Port=10
  out09 : Outport  Port=11
  out010 : Outport  Port=12
  out011 : Outport  Port=13
  out012 : Outport  Port=14
  out013 : Outport  Port=15
  out014 : Outport  Port=16
  out015 : Outport  Port=17
  of : Outport  Port=18
  wires:
  in00/1 -> abus/1(in1)
  adebus3_0/1(msb_lsb_out1) -> out00/1
  in01/1 -> abus/2(in2)
  adebus3_4/1(msb_lsb_out1) -> out01/1
  in02/1 -> abus/3(in3)
  adebus3_2/1(msb_lsb_out1) -> out02/1
  in03/1 -> abus/4(in4)
  adebus3_6/1(msb_lsb_out1) -> out03/1
  in04/1 -> abus/5(in5)
  adebus3_1/1(msb_lsb_out1) -> out04/1
  in05/1 -> abus/6(in6)
  adebus3_5/1(msb_lsb_out1) -> out05/1
  in06/1 -> abus/7(in7)
  adebus3_3/1(msb_lsb_out1) -> out06/1
  in07/1 -> abus/8(in8)
  adebus3_7/1(msb_lsb_out1) -> out07/1
  in08/1 -> bbus/1(in1)
  bdebus3_0/1(msb_lsb_out1) -> out08/1
  in09/1 -> bbus/2(in2)
  bdebus3_4/1(msb_lsb_out1) -> out09/1
  in010/1 -> bbus/3(in3)
  bdebus3_2/1(msb_lsb_out1) -> out010/1
  in011/1 -> bbus/4(in4)
  bdebus3_6/1(msb_lsb_out1) -> out011/1
  in012/1 -> bbus/5(in5)
  bdebus3_1/1(msb_lsb_out1) -> out012/1
  in013/1 -> bbus/6(in6)
  bdebus3_5/1(msb_lsb_out1) -> out013/1
  in014/1 -> bbus/7(in7)
  bdebus3_3/1(msb_lsb_out1) -> out014/1
  in015/1 -> bbus/8(in8)
  bdebus3_7/1(msb_lsb_out1) -> out015/1
  of_or/1 -> of_expand/1(bus_in)
  of_expand/1(msb_out8) -> combine/1
  of_expand/2(out7) -> combine/2
  of_expand/3(out6) -> combine/3
  of_expand/4(out5) -> combine/4
  of_expand/5(out4) -> combine/5
  of_expand/6(out3) -> combine/6
  of_expand/7(out2) -> combine/7
  of_expand/8(lsb_out1) -> combine/8
  combine/1 -> of/1
  shift/1 -> slice0/1, slice1/1, slice2/1, slice3/1
  of_0/1 -> of_or/1
  sync/1 -> butterfly0_0/3(sync_in)
  slice0/1 -> butterfly0_0/4(shift)
  abus/1(bus_out) -> butterfly0_0/1(a)
  bbus/1(bus_out) -> butterfly0_0/2(b)
  butterfly0_0/3(of) -> of_0/1
  butterfly0_0/1(a+bw) -> adebus0_0/1(bus_in)
  butterfly0_0/2(a-bw) -> bdebus0_0/1(bus_in)
  of_1/1 -> of_or/2
  butterfly0_0/4(sync_out) -> butterfly1_0/3(sync_in), butterfly1_1/3(sync_in)
  slice1/1 -> butterfly1_0/4(shift), butterfly1_1/4(shift)
  adebus0_0/1(msb_out2) -> butterfly1_0/1(a)
  adebus0_0/2(lsb_out1) -> butterfly1_0/2(b)
  butterfly1_0/3(of) -> of_1/1
  butterfly1_0/1(a+bw) -> adebus1_0/1(bus_in)
  butterfly1_0/2(a-bw) -> bdebus1_0/1(bus_in)
  bdebus0_0/1(msb_out2) -> butterfly1_1/1(a)
  bdebus0_0/2(lsb_out1) -> butterfly1_1/2(b)
  butterfly1_1/3(of) -> of_1/2
  butterfly1_1/1(a+bw) -> adebus1_1/1(bus_in)
  butterfly1_1/2(a-bw) -> bdebus1_1/1(bus_in)
  of_2/1 -> of_or/3
  butterfly1_0/4(sync_out) -> butterfly2_0/3(sync_in), butterfly2_1/3(sync_in)
  slice2/1 -> butterfly2_0/4(shift), butterfly2_1/4(shift), butterfly2_2/4(shift), butterfly2_3/4(shift)
  adebus1_0/1(msb_out2) -> butterfly2_0/1(a)
  adebus1_0/2(lsb_out1) -> butterfly2_0/2(b)
  butterfly2_0/3(of) -> of_2/1
  butterfly2_0/1(a+bw) -> adebus2_0/1(bus_in)
  butterfly2_0/2(a-bw) -> bdebus2_0/1(bus_in)
  bdebus1_0/1(msb_out2) -> butterfly2_1/1(a)
  bdebus1_0/2(lsb_out1) -> butterfly2_1/2(b)
  butterfly2_1/3(of) -> of_2/2
  butterfly2_1/1(a+bw) -> adebus2_1/1(bus_in)
  butterfly2_1/2(a-bw) -> bdebus2_1/1(bus_in)
  butterfly1_1/4(sync_out) -> butterfly2_2/3(sync_in), butterfly2_3/3(sync_in)
  adebus1_1/1(msb_out2) -> butterfly2_2/1(a)
  adebus1_1/2(lsb_out1) -> butterfly2_2/2(b)
  butterfly2_2/3(of) -> of_2/3
  butterfly2_2/1(a+bw) -> adebus2_2/1(bus_in)
  butterfly2_2/2(a-bw) -> bdebus2_2/1(bus_in)
  bdebus1_1/1(msb_out2) -> butterfly2_3/1(a)
  bdebus1_1/2(lsb_out1) -> butterfly2_3/2(b)
  butterfly2_3/3(of) -> of_2/4
  butterfly2_3/1(a+bw) -> adebus2_3/1(bus_in)
  butterfly2_3/2(a-bw) -> bdebus2_3/1(bus_in)
  of_3/1 -> of_or/4
  butterfly2_0/4(sync_out) -> butterfly3_0/3(sync_in), butterfly3_1/3(sync_in)
  butterfly3_0/4(sync_out) -> sync_out/1
  slice3/1 -> butterfly3_0/4(shift), butterfly3_1/4(shift), butterfly3_2/4(shift), butterfly3_3/4(shift), butterfly3_4/4(shift), butterfly3_5/4(shift), butterfly3_6/4(shift), butterfly3_7/4(shift)
  adebus2_0/1(msb_out2) -> butterfly3_0/1(a)
  adebus2_0/2(lsb_out1) -> butterfly3_0/2(b)
  butterfly3_0/3(of) -> of_3/1
  butterfly3_1/4(sync_out) -> synct1/1
  bdebus2_0/1(msb_out2) -> butterfly3_1/1(a)
  bdebus2_0/2(lsb_out1) -> butterfly3_1/2(b)
  butterfly3_1/3(of) -> of_3/2
  butterfly2_1/4(sync_out) -> butterfly3_2/3(sync_in), butterfly3_3/3(sync_in)
  butterfly3_2/4(sync_out) -> synct2/1
  adebus2_1/1(msb_out2) -> butterfly3_2/1(a)
  adebus2_1/2(lsb_out1) -> butterfly3_2/2(b)
  butterfly3_2/3(of) -> of_3/3
  butterfly3_3/4(sync_out) -> synct3/1
  bdebus2_1/1(msb_out2) -> butterfly3_3/1(a)
  bdebus2_1/2(lsb_out1) -> butterfly3_3/2(b)
  butterfly3_3/3(of) -> of_3/4
  butterfly2_2/4(sync_out) -> butterfly3_4/3(sync_in), butterfly3_5/3(sync_in)
  butterfly3_4/4(sync_out) -> synct4/1
  adebus2_2/1(msb_out2) -> butterfly3_4/1(a)
  adebus2_2/2(lsb_out1) -> butterfly3_4/2(b)
  butterfly3_4/3(of) -> of_3/5
  butterfly3_5/4(sync_out) -> synct5/1
  bdebus2_2/1(msb_out2) -> butterfly3_5/1(a)
  bdebus2_2/2(lsb_out1) -> butterfly3_5/2(b)
  butterfly3_5/3(of) -> of_3/6
  butterfly2_3/4(sync_out) -> butterfly3_6/3(sync_in), butterfly3_7/3(sync_in)
  butterfly3_6/4(sync_out) -> synct6/1
  adebus2_3/1(msb_out2) -> butterfly3_6/1(a)
  adebus2_3/2(lsb_out1) -> butterfly3_6/2(b)
  butterfly3_6/3(of) -> of_3/7
  butterfly3_7/4(sync_out) -> synct7/1
  bdebus2_3/1(msb_out2) -> butterfly3_7/1(a)
  bdebus2_3/2(lsb_out1) -> butterfly3_7/2(b)
  butterfly3_7/3(of) -> of_3/8
  butterfly3_0/1(a+bw) -> adebus3_0/1(bus_in)
  butterfly3_0/2(a-bw) -> bdebus3_0/1(bus_in)
  butterfly3_1/1(a+bw) -> adebus3_1/1(bus_in)
  butterfly3_1/2(a-bw) -> bdebus3_1/1(bus_in)
  butterfly3_2/1(a+bw) -> adebus3_2/1(bus_in)
  butterfly3_2/2(a-bw) -> bdebus3_2/1(bus_in)
  butterfly3_3/1(a+bw) -> adebus3_3/1(bus_in)
  butterfly3_3/2(a-bw) -> bdebus3_3/1(bus_in)
  butterfly3_4/1(a+bw) -> adebus3_4/1(bus_in)
  butterfly3_4/2(a-bw) -> bdebus3_4/1(bus_in)
  butterfly3_5/1(a+bw) -> adebus3_5/1(bus_in)
  butterfly3_5/2(a-bw) -> bdebus3_5/1(bus_in)
  butterfly3_6/1(a+bw) -> adebus3_6/1(bus_in)
  butterfly3_6/2(a-bw) -> bdebus3_6/1(bus_in)
  butterfly3_7/1(a+bw) -> adebus3_7/1(bus_in)
  butterfly3_7/2(a-bw) -> bdebus3_7/1(bus_in)
